simulink model "WheelLoads"  (R2020b)
settings: Solver=ode23  StartTime=0.0  StopTime=5  RelTol=0.01  AbsTol=0.01
PreLoadFcn:
  | addpath('Functions');
  | addpath('Functions/geometric');
  | addpath('Parameters');
  | vhclParams;
  | 
  | ELLIPSE = Simulink.Variant('variantMode == 1');
  | CONSTANT_ACC = Simulink.Variant('variantMode == 2');
  | CONSTANT_OUT = Simulink.Variant('variantMode == 3');
  | EXTERNAL_DATA_LOAD = Simulink.Variant('variantMode == 4');
  | EXTERNAL_DATA_ACC = Simulink.Variant('variantMode == 5');
  | if ~exist('F','var')
  |  F = 0;
  | end
  | if ~exist('M','var')
  |  M = 0;
  | end
  | if ~exist('St','var')
  |  St = 0;
  | end
  | if ~exist('Ax','var')
  |  Ax = 0;
  | end
  | if ~exist('Ay','var')
  |  Ay = 0;
  | end
  | if ~exist('StAcc','var')
  |  StAcc = 0;
  | end
InitFcn:
  | [folder, ~, ~] = fileparts(which (bdroot));
  | cd(folder);
undefined symbols (not defined in the file): ARB_bcrnk_frontL, ARB_bcrnk_frontR, ARB_bcrnk_rearL, ARB_bcrnk_rearR, ARB_center_front, ARB_center_rear, ARB_damping_front, ARB_damping_rear, ARB_length_front, ARB_length_rear, ARB_pinSlot_correctionFactor_front, ARB_pinSlot_correctionFactor_rear, ARB_stiffness_front, ARB_stiffness_rear, ARB_TieRod_length_front, ARB_TieRod_length_rear, COG, Damper_coefficient_front, Damper_coefficient_rear, Driver_COG, Driver_mass, DriveTrain_ratio, FS_camber, FS_toe, gravity, Mass, Rim_mass, RodDiameter, RodThickness, RS_camber, RS_toe, sep_lower_front, sep_lower_rear, sep_upper_front, sep_upper_rear, Spring_preload_front, Spring_preload_rear, Spring_stiffness_front, Spring_stiffness_rear, Sprung_mass, Sprung_mass_COG, sym_lower_front, sym_lower_rear, sym_upper_front, sym_upper_rear, Tire_mass, Tire_radius, Unsprung_mass_COG_front, Unsprung_mass_COG_rear, Unsprung_mass_front, Unsprung_mass_rear, util_rodCrossSection, Wheel_inertia, X, X_F, X_R, Y, Z
libraries: sm_lib x404, nesl_utility x74, fl_ps_legacy_lib x1
(part 3 of 18)

== system WheelLoads/Load actuator/Constant acc ==
  Bus Creator : BusCreator  Inputs=2
  Constant : Constant  Value=0
  From Workspace : FromWorkspace  VariableName=Fx
  From Workspace1 : FromWorkspace  VariableName=Ax
  From Workspace2 : FromWorkspace  VariableName=Fy
  From Workspace3 : FromWorkspace  VariableName=Fz
  From Workspace4 : FromWorkspace  VariableName=Ay
  From Workspace6 : FromWorkspace  VariableName=St
  Matrix Concatenate : Concatenate  NumInputs=3  Mode=Multidimensional array  ConcatenateDimension=2
  Matrix Concatenate1 : Concatenate  NumInputs=3  Mode=Multidimensional array  ConcatenateDimension=2
  Saturation : Saturate  UpperLimit=21*driveRatio/tireR  LowerLimit=-inf
  Terminator : Terminator
  Vector Concatenate : Concatenate  NumInputs=4
  M : Outport
  StrAng : Outport  Port=2
  F : Outport  Port=3
  wires:
  From Workspace6/1 -> StrAng/1
  Matrix Concatenate1/1 -> M/1
  Constant/1 -> Vector Concatenate/3, Vector Concatenate/4, Vector Concatenate/1, Vector Concatenate/2
  Vector Concatenate/1 -> Matrix Concatenate1/3, Matrix Concatenate1/1, Matrix Concatenate1/2
  From Workspace4/1 -> Bus Creator/2  "ay"
  From Workspace1/1 -> Bus Creator/1  "ax"
  Bus Creator/1 -> Terminator/1  "a"
  Saturation/1 -> Matrix Concatenate/1
  From Workspace/1 -> Saturation/1
  From Workspace2/1 -> Matrix Concatenate/2
  From Workspace3/1 -> Matrix Concatenate/3
  Matrix Concatenate/1 -> F/1

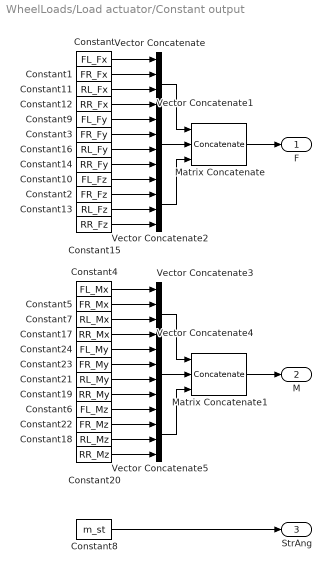
== system WheelLoads/Load actuator/Constant output ==
  Constant : Constant  Value=FL_Fx
  Constant1 : Constant  Value=FR_Fx
  Constant10 : Constant  Value=FL_Fz
  Constant11 : Constant  Value=RL_Fx
  Constant12 : Constant  Value=RR_Fx
  Constant13 : Constant  Value=RL_Fz
  Constant14 : Constant  Value=RR_Fy  VectorParams1D=off
  Constant15 : Constant  Value=RR_Fz
  Constant16 : Constant  Value=RL_Fy
  Constant17 : Constant  Value=RR_Mx
  Constant18 : Constant  Value=RL_Mz
  Constant19 : Constant  Value=RR_My  VectorParams1D=off
  Constant2 : Constant  Value=FR_Fz
  Constant20 : Constant  Value=RR_Mz
  Constant21 : Constant  Value=RL_My
  Constant22 : Constant  Value=FR_Mz
  Constant23 : Constant  Value=FR_My
  Constant24 : Constant  Value=FL_My
  Constant3 : Constant  Value=FR_Fy
  Constant4 : Constant  Value=FL_Mx
  Constant5 : Constant  Value=FR_Mx
  Constant6 : Constant  Value=FL_Mz
  Constant7 : Constant  Value=RL_Mx
  Constant8 : Constant  Value=m_st
  Constant9 : Constant  Value=FL_Fy
  Matrix Concatenate : Concatenate  NumInputs=3  Mode=Multidimensional array  ConcatenateDimension=2
  Matrix Concatenate1 : Concatenate  NumInputs=3  Mode=Multidimensional array  ConcatenateDimension=2
  Vector Concatenate{,1..5} (6 alike) : Concatenate  NumInputs=4
  F : Outport
  M : Outport  Port=2
  StrAng : Outport  Port=3
  wires:
  Constant8/1 -> StrAng/1
  Vector Concatenate5/1 -> Matrix Concatenate1/3
  Vector Concatenate3/1 -> Matrix Concatenate1/1
  Vector Concatenate4/1 -> Matrix Concatenate1/2
  Constant4/1 -> Vector Concatenate3/1
  Constant5/1 -> Vector Concatenate3/2
  Constant7/1 -> Vector Concatenate3/3
  Constant17/1 -> Vector Concatenate3/4
  Constant24/1 -> Vector Concatenate4/1
  Constant23/1 -> Vector Concatenate4/2
  Constant21/1 -> Vector Concatenate4/3
  Constant19/1 -> Vector Concatenate4/4
  Constant6/1 -> Vector Concatenate5/1
  Constant22/1 -> Vector Concatenate5/2
  Constant18/1 -> Vector Concatenate5/3
  Constant20/1 -> Vector Concatenate5/4
  Constant15/1 -> Vector Concatenate2/4
  Constant13/1 -> Vector Concatenate2/3
  Constant2/1 -> Vector Concatenate2/2
  Constant10/1 -> Vector Concatenate2/1
  Constant14/1 -> Vector Concatenate1/4
  Vector Concatenate2/1 -> Matrix Concatenate/3
  Vector Concatenate1/1 -> Matrix Concatenate/2
  Constant16/1 -> Vector Concatenate1/3
  Constant3/1 -> Vector Concatenate1/2
  Constant9/1 -> Vector Concatenate1/1
  Constant12/1 -> Vector Concatenate/4
  Constant11/1 -> Vector Concatenate/3
  Constant1/1 -> Vector Concatenate/2
  Constant/1 -> Vector Concatenate/1
  Matrix Concatenate/1 -> F/1
  Matrix Concatenate1/1 -> M/1
  Vector Concatenate/1 -> Matrix Concatenate/1

== system WheelLoads/Load actuator/Ellipse ==
  Bus Creator : BusCreator  Inputs=2
  Constant : Constant  Value=0
  From Workspace : FromWorkspace  VariableName=Fx
  From Workspace1 : FromWorkspace  VariableName=Ax
  From Workspace2 : FromWorkspace  VariableName=Fy
  From Workspace3 : FromWorkspace  VariableName=Fz
  From Workspace4 : FromWorkspace  VariableName=Ay
  From Workspace6 : FromWorkspace  VariableName=St
  Matrix Concatenate : Concatenate  NumInputs=3  Mode=Multidimensional array  ConcatenateDimension=2
  Matrix Concatenate1 : Concatenate  NumInputs=3  Mode=Multidimensional array  ConcatenateDimension=2
  Saturation : Saturate  UpperLimit=21*driveRatio/tireR  LowerLimit=-inf
  Terminator : Terminator
  Vector Concatenate : Concatenate  NumInputs=4
  F : Outport
  M : Outport  Port=2
  StrAng : Outport  Port=3
  wires:
  From Workspace6/1 -> StrAng/1
  Vector Concatenate/1 -> Matrix Concatenate1/2, Matrix Concatenate1/1, Matrix Concatenate1/3
  Constant/1 -> Vector Concatenate/2, Vector Concatenate/1, Vector Concatenate/4, Vector Concatenate/3
  Matrix Concatenate1/1 -> M/1
  Matrix Concatenate/1 -> F/1
  From Workspace3/1 -> Matrix Concatenate/3
  From Workspace/1 -> Saturation/1
  From Workspace2/1 -> Matrix Concatenate/2
  From Workspace1/1 -> Bus Creator/1  "ax"
  From Workspace4/1 -> Bus Creator/2  "ay"
  Bus Creator/1 -> Terminator/1  "a"
  Saturation/1 -> Matrix Concatenate/1

== system WheelLoads/Load actuator/External data - acc ==
  Bus Creator : BusCreator  Inputs=2
  Constant : Constant  Value=0
  From Workspace : FromWorkspace  VariableName=Fx
  From Workspace1 : FromWorkspace  VariableName=ext_ax
  From Workspace2 : FromWorkspace  VariableName=Fy
  From Workspace3 : FromWorkspace  VariableName=Fz
  From Workspace4 : FromWorkspace  VariableName=ext_ay
  From Workspace6 : FromWorkspace  VariableName=St
  Matrix Concatenate : Concatenate  NumInputs=3  Mode=Multidimensional array  ConcatenateDimension=2
  Matrix Concatenate1 : Concatenate  NumInputs=3  Mode=Multidimensional array  ConcatenateDimension=2
  Saturation : Saturate  UpperLimit=21*driveRatio/tireR  LowerLimit=-inf
  Terminator : Terminator
  Vector Concatenate : Concatenate  NumInputs=4
  M : Outport
  StrAng : Outport  Port=2
  F : Outport  Port=3
  wires:
  Matrix Concatenate1/1 -> M/1
  Constant/1 -> Vector Concatenate/3, Vector Concatenate/4, Vector Concatenate/1, Vector Concatenate/2
  Vector Concatenate/1 -> Matrix Concatenate1/3, Matrix Concatenate1/1, Matrix Concatenate1/2
  From Workspace6/1 -> StrAng/1
  Bus Creator/1 -> Terminator/1  "a"
  From Workspace1/1 -> Bus Creator/1  "ax"
  From Workspace4/1 -> Bus Creator/2  "ay"
  Saturation/1 -> Matrix Concatenate/1
  From Workspace/1 -> Saturation/1
  From Workspace2/1 -> Matrix Concatenate/2
  From Workspace3/1 -> Matrix Concatenate/3
  Matrix Concatenate/1 -> F/1

== system WheelLoads/Load actuator/External data - load ==
  From Workspace : FromWorkspace  VariableName=F.Fx
  From Workspace1 : FromWorkspace  VariableName=M.Mx
  From Workspace2 : FromWorkspace  VariableName=F.Fy
  From Workspace3 : FromWorkspace  VariableName=F.Fz
  From Workspace4 : FromWorkspace  VariableName=M.My
  From Workspace5 : FromWorkspace  VariableName=M.Mz
  From Workspace6 : FromWorkspace  VariableName=St
  Matrix Concatenate : Concatenate  NumInputs=3  Mode=Multidimensional array  ConcatenateDimension=2
  Matrix Concatenate1 : Concatenate  NumInputs=3  Mode=Multidimensional array  ConcatenateDimension=2
  F : Outport
  M : Outport  Port=2
  StrAng : Outport  Port=3
  wires:
  From Workspace6/1 -> StrAng/1
  Matrix Concatenate1/1 -> M/1
  From Workspace1/1 -> Matrix Concatenate1/1
  From Workspace4/1 -> Matrix Concatenate1/2
  From Workspace5/1 -> Matrix Concatenate1/3
  From Workspace3/1 -> Matrix Concatenate/3
  From Workspace2/1 -> Matrix Concatenate/2
  From Workspace/1 -> Matrix Concatenate/1
  Matrix Concatenate/1 -> F/1
